simulink model "TosAeb"  (R2021a)
settings: Solver=FixedStepDiscrete  StartTime=0.0  StopTime=inf  FixedStep=0.01  RelTol=1e-3
undefined symbols (not defined in the file or in its folder): ACCModSts, AccSts1, AEB_ObjectMovingOrientation, AEB_ObjectMovingState, CN_BTCActive, CN_CurrentGearR, CN_CurrentGearR_DHT, CN_PTCActive, CN_VDCActive, CN_VSC_EspOff, CN_VSC_FCW_AEB_Error, CN_VSC_FCW_AEB_NoDispay, CN_VSC_FCW_AEB_PerfmDgdtn, CN_VSC_FCW_AEB_TempUnAvlbl, CN_VSC_VehStandstill, CNaccelAvoidMax, CNaccelAvoidMin, CNbrakePedalPress, CNcomfortStrLeftAccelMax, CNcomfortStrLeftAccelMin, CNcomfortStrRightAccelMax, CNcomfortStrRightAccelMin, CNDrvDoorOpen, CNDrvModeEco, CNDrvModeNorm, CNDrvModeOther, CNDrvModeSport, CNDrvSBRreminder, CNNull, CNobjInvalidID, CNPassDoorOpen, CNPassSBRreminder, CNPEBSAccelNegInf, CNPEBSAccelNull, CNPEBSTimeInf, CNPEBSTimeNull, CNSTOPMAX, CNSTOPNULL, CNsubjWidth, CNSwtOFF, CNSwtON, CNTTCMAX, CNTTCNULL, CNVehConfigAEBCycNone, CNVehConfigAEBNone, CNVehConfigAEBPedNone, CNVehConfigFCWNone, CNVehConfigJANone, CollisionSeverity, FALSE, FCWSnvty, JACycObjCalcInfoDefaultParams, JAPedObjCalcInfoDefaultParams, JAVehObjCalcInfoDefaultParams, latCycObjCalcInfoDefaultParams, latPedObjCalcInfoDefaultParams, longObjCalcInfoDefaultParams, ModSts, movingStatus, ObjectType ... (+1112)
libraries: simulink x3031, AEBSFunctionLib x50, Ta_Library x33, AEBSStateMachineLib x23, Mylibrary x12, simulink_extras x7, ObjSelect_lib x3, dspstat3 x2
(part 98 of 102)

== stateflow chart TosAeb/FcwAebJa_Step_sys/ALG_AEBSystem/ALG_AEBController/AEB_LongTgtInfoCalc/LongObjCalcInfoCalc/AEBS_LongCollisionAssesApp/TTCCalc/TTCCalc/Situation2/SloveTTCQuadraticEquation/AccelRelUnequalZero/DeltaGreaterEqualZero/RawTTCCalcu ==
  (default) -> (junction 11)
  (junction 11) -> (junction 12) [order 1] : [TTC1 < 0 && TTC2 < 0]
  (junction 11) -> (junction 13) [order 2]
  (junction 12) -> (junction 17) : {
      | 	TTC = CNNull;
      | }
  (junction 13) -> (junction 14) [order 1] : [TTC1 > 0 && TTC2 > 0]
  (junction 13) -> (junction 15) [order 2] : {
      | 	TTC = max(TTC1,TTC2);
      | }
  (junction 14) -> (junction 16) : {
      | 	TTC = min(TTC1,TTC2);
      | }
  (junction 16) -> (junction 15)
  (junction 17) -> (junction 16)
  (junction 15) -> (junction 18)
  data: TTC1 input; TTC2 input; TTC output; CNNull constant double

== stateflow chart TosAeb/FcwAebJa_Step_sys/ALG_AEBSystem/ALG_AEBController/AEB_LongTgtInfoCalc/LongObjCalcInfoCalc/AEBS_LongCollisionAssesApp/TTCCalc/TTCCalc/Situation3/SloveTTCQuadraticEquation/AccelRelEqualsZero/Chart ==
  state tempTTC = TTCCalc(distRel,velRel) [function]
  (default) -> (junction 17)
  (junction 17) -> (junction 18) [order 1] : [velRel >= 0 && distRel >= 0]
  (junction 17) -> (junction 19) [order 2]
  (junction 18) -> (junction 23) : {
      | 	TTC = CNSTOPMAX;
      | }
  (junction 19) -> (junction 20) [order 1] : [velRel <= 0 && distRel <= 0]
  (junction 19) -> (junction 21) [order 2] : {
      | 	 TTC = TTCCalc(distRel,velRel);
      | }
  (junction 20) -> (junction 22) : {
      | 	TTC = CNNull;
      | }
  (junction 22) -> (junction 21)
  (junction 23) -> (junction 22)
  (junction 21) -> (junction 24)
  data: distRel function_input; velRel function_input; tempTTC function_output; TTC output; CNSTOPMAX parameter; CNNull parameter; distRel input; velRel input

== stateflow chart TosAeb/FcwAebJa_Step_sys/ALG_AEBSystem/ALG_AEBController/AEB_LongTgtInfoCalc/LongObjCalcInfoCalc/AEBS_LongCollisionAssesApp/TTCCalc/TTCCalc/Situation3/SloveTTCQuadraticEquation/AccelRelUnequalZero/DeltaGreaterEqualZero/RawTTCCalcu ==
  (default) -> (junction 11)
  (junction 11) -> (junction 12) [order 1] : [TTC1 < 0 && TTC2 < 0]
  (junction 11) -> (junction 13) [order 2]
  (junction 12) -> (junction 17) : {
      | 	TTC = CNNull;
      | }
  (junction 13) -> (junction 14) [order 1] : [TTC1 > 0 && TTC2 > 0]
  (junction 13) -> (junction 15) [order 2] : {
      | 	TTC = max(TTC1,TTC2);
      | }
  (junction 14) -> (junction 16) : {
      | 	TTC = min(TTC1,TTC2);
      | }
  (junction 16) -> (junction 15)
  (junction 17) -> (junction 16)
  (junction 15) -> (junction 18)
  data: TTC1 input; TTC2 input; TTC output; CNNull constant double

== stateflow chart TosAeb/FcwAebJa_Step_sys/ALG_AEBSystem/ALG_AEBController/AEB_LongTgtInfoCalc/LongObjCalcInfoCalc/AEBS_LongCollisionAssesApp/TTCCalc/TTCCalc/SloveTTCQuadraticEquation/AccelRelEqualsZero/Chart ==
  state tempTTC = TTCCalc(distRel,velRel) [function]
  (default) -> (junction 17)
  (junction 17) -> (junction 18) [order 1] : [velRel >= 0 && distRel >= 0]
  (junction 17) -> (junction 19) [order 2]
  (junction 18) -> (junction 23) : {
      | 	TTC = CNSTOPMAX;
      | }
  (junction 19) -> (junction 20) [order 1] : [velRel <= 0 && distRel <= 0]
  (junction 19) -> (junction 21) [order 2] : {
      | 	 TTC = TTCCalc(distRel,velRel);
      | }
  (junction 20) -> (junction 22) : {
      | 	TTC = CNNull;
      | }
  (junction 22) -> (junction 21)
  (junction 23) -> (junction 22)
  (junction 21) -> (junction 24)
  data: distRel function_input; velRel function_input; tempTTC function_output; TTC output; CNSTOPMAX parameter; CNNull parameter; distRel input; velRel input

== stateflow chart TosAeb/FcwAebJa_Step_sys/ALG_AEBSystem/ALG_AEBController/AEB_LongTgtInfoCalc/LongObjCalcInfoCalc/AEBS_LongCollisionAssesApp/TTCCalc/TTCCalc/SloveTTCQuadraticEquation/AccelRelUnequalZero/DeltaGreaterEqualZero/RawTTCCalcu ==
  (default) -> (junction 11)
  (junction 11) -> (junction 12) [order 1] : [TTC1 < 0 && TTC2 < 0]
  (junction 11) -> (junction 13) [order 2]
  (junction 12) -> (junction 17) : {
      | 	TTC = CNNull;
      | }
  (junction 13) -> (junction 14) [order 1] : [TTC1 > 0 && TTC2 > 0]
  (junction 13) -> (junction 15) [order 2] : {
      | 	TTC = max(TTC1,TTC2);
      | }
  (junction 14) -> (junction 16) : {
      | 	TTC = min(TTC1,TTC2);
      | }
  (junction 16) -> (junction 15)
  (junction 17) -> (junction 16)
  (junction 15) -> (junction 18)
  data: TTC1 input; TTC2 input; TTC output; CNNull constant double

== stateflow chart TosAeb/FcwAebJa_Step_sys/ALG_AEBSystem/ALG_AEBController/AEB_LongTgtInfoCalc/LongObjCalcInfoCalc/AEBS_LongCollisionAssesApp/TTCCalc/TTCCalc/TTCCalc ==
  (default) -> (junction 17)
  (junction 17) -> (junction 18) [order 1] : [rawTTC < tEgoStop &&...
      | rawTTC < tObjStop]
  (junction 17) -> (junction 19) [order 2]
  (junction 18) -> (junction 23) : {TTC = rawTTC;}
  (junction 19) -> (junction 20) [order 1] : [rawTTC > tEgoStop &&...
      |  rawTTC < tObjStop]
  (junction 19) -> (junction 26) [order 2]
  (junction 20) -> (junction 22) : {
      | 	send(situation2);
      | 	TTC = TTCIn1;
      | }
  (junction 26) -> (junction 27) [order 1] : [rawTTC > tObjStop &&...
      |  rawTTC < tEgoStop]
  (junction 26) -> (junction 21) [order 2] : {TTC = CNTTCMAX;}
  (junction 27) -> (junction 29) : {
      | 	send(situation3);
      | 	TTC = TTCIn2;
      | }
  (junction 29) -> (junction 21)
  (junction 22) -> (junction 29)
  (junction 23) -> (junction 22)
  (junction 21) -> (junction 25)
  data: tEgoStop input; tObjStop input; rawTTC input; TTC output single; CNTTCMAX parameter single; TTCIn1 input; TTCIn2 input
  events: situation2 output FUNCTION_CALL_EVENT; situation3 output FUNCTION_CALL_EVENT

== stateflow chart TosAeb/FcwAebJa_Step_sys/ALG_AEBSystem/ALG_AEBController/AEB_LongTgtInfoCalc/LongObjCalcInfoCalc/AEBS_LongCollisionAssesApp/TTCCalc/TTCCalc/tEgoStopCalc/StopTimeFlow ==
  state temptStop = tStopFuncCalc(velRel,accelRel) [function]
  (default) -> (junction 2)
  (junction 2) -> (junction 3) [order 1] : [accelRel == 0]
  (junction 2) -> (junction 14) [order 2]
  (junction 3) -> (junction 5)
  (junction 14) -> (junction 16) [order 1] : [(accelRel > 0 && velRel <= 0)||...
      | (accelRel < 0 && velRel >= 0)]
  (junction 5) -> (junction 7) [order 1] : [velRel == 0]
  (junction 14) -> (junction 20) [order 2] : {
      | 	tStop = CNSTOPMAX;
      |  }
  (junction 16) -> (junction 18) : {
      |
      | 	tStop = tStopFuncCalc(velRel,accelRel);
      | }
  (junction 5) -> (junction 11) [order 2] : {
      | 	tStop = CNSTOPMAX;
      | }
  (junction 7) -> (junction 9) : {
      | 	tStop = CNNull;
      | }
  (junction 18) -> (junction 20)
  (junction 11) -> (junction 18)
  (junction 9) -> (junction 11)
  (junction 20) -> (junction 24)
  data: velRel function_input; accelRel function_input; temptStop function_output; tStop output; CNNull parameter double; CNSTOPMAX parameter double; accelRel input; velRel input

== stateflow chart TosAeb/FcwAebJa_Step_sys/ALG_AEBSystem/ALG_AEBController/AEB_LongTgtInfoCalc/LongObjCalcInfoCalc/AEBS_LongCollisionAssesApp/TTCCalc/TTCCalc/tObjStopCalc/StopTimeFlow ==
  state temptStop = tStopFuncCalc(velRel,accelRel) [function]
  (default) -> (junction 2)
  (junction 2) -> (junction 3) [order 1] : [accelRel == 0]
  (junction 2) -> (junction 14) [order 2]
  (junction 3) -> (junction 5)
  (junction 14) -> (junction 16) [order 1] : [(accelRel > 0 && velRel <= 0)||...
      | (accelRel < 0 && velRel >= 0)]
  (junction 5) -> (junction 7) [order 1] : [velRel == 0]
  (junction 14) -> (junction 20) [order 2] : {
      | 	tStop = CNSTOPMAX;
      |  }
  (junction 16) -> (junction 18) : {
      |
      | 	tStop = tStopFuncCalc(velRel,accelRel);
      | }
  (junction 5) -> (junction 11) [order 2] : {
      | 	tStop = CNSTOPMAX;
      | }
  (junction 7) -> (junction 9) : {
      | 	tStop = CNNull;
      | }
  (junction 18) -> (junction 20)
  (junction 11) -> (junction 18)
  (junction 9) -> (junction 11)
  (junction 20) -> (junction 24)
  data: velRel function_input; accelRel function_input; temptStop function_output; tStop output; CNNull parameter double; CNSTOPMAX parameter double; accelRel input; velRel input

== stateflow chart TosAeb/FcwAebJa_Step_sys/ALG_AEBSystem/ALG_AEBController/AEB_LongTgtInfoCalc/LongObjCalcInfoCalc/AEBS_LongCollisionAssesApp/collisionSeverityHighCalc/collisionSeverityHighCalc1/CollisionAssessHighCalcVersion3/CollisionAssessHighCalc1/CalcBrakePhase1/tEgoStopCalc/tStopCalc/StopTimeFlow ==
  state temptStop = tStopFuncCalc(velRel,accelRel) [function]
  (default) -> (junction 2)
  (junction 2) -> (junction 3) [order 1] : [accelRel == 0]
  (junction 2) -> (junction 14) [order 2]
  (junction 3) -> (junction 5)
  (junction 14) -> (junction 16) [order 1] : [(accelRel > 0 && velRel <= 0)||...
      | (accelRel < 0 && velRel >= 0)]
  (junction 5) -> (junction 7) [order 1] : [velRel == 0]
  (junction 14) -> (junction 20) [order 2] : {
      | 	tStop = CNSTOPMAX;
      |  }
  (junction 16) -> (junction 18) : {
      |
      | 	tStop = tStopFuncCalc(velRel,accelRel);
      | }
  (junction 5) -> (junction 11) [order 2] : {
      | 	tStop = CNSTOPMAX;
      | }
  (junction 7) -> (junction 9) : {
      | 	tStop = CNNull;
      | }
  (junction 18) -> (junction 20)
  (junction 11) -> (junction 18)
  (junction 9) -> (junction 11)
  (junction 20) -> (junction 24)
  data: velRel function_input; accelRel function_input; temptStop function_output; tStop output; CNNull parameter double; CNSTOPMAX parameter double; accelRel input; velRel input

== stateflow chart TosAeb/FcwAebJa_Step_sys/ALG_AEBSystem/ALG_AEBController/AEB_LongTgtInfoCalc/LongObjCalcInfoCalc/AEBS_LongCollisionAssesApp/collisionSeverityHighCalc/collisionSeverityHighCalc1/CollisionAssessHighCalcVersion3/CollisionAssessHighCalc1/CalcBrakePhase1/tObjStopCalc/tStopCalc/StopTimeFlow ==
  state temptStop = tStopFuncCalc(velRel,accelRel) [function]
  (default) -> (junction 2)
  (junction 2) -> (junction 3) [order 1] : [accelRel == 0]
  (junction 2) -> (junction 14) [order 2]
  (junction 3) -> (junction 5)
  (junction 14) -> (junction 16) [order 1] : [(accelRel > 0 && velRel <= 0)||...
      | (accelRel < 0 && velRel >= 0)]
  (junction 5) -> (junction 7) [order 1] : [velRel == 0]
  (junction 14) -> (junction 20) [order 2] : {
      | 	tStop = CNSTOPMAX;
      |  }
  (junction 16) -> (junction 18) : {
      |
      | 	tStop = tStopFuncCalc(velRel,accelRel);
      | }
  (junction 5) -> (junction 11) [order 2] : {
      | 	tStop = CNSTOPMAX;
      | }
  (junction 7) -> (junction 9) : {
      | 	tStop = CNNull;
      | }
  (junction 18) -> (junction 20)
  (junction 11) -> (junction 18)
  (junction 9) -> (junction 11)
  (junction 20) -> (junction 24)
  data: velRel function_input; accelRel function_input; temptStop function_output; tStop output; CNNull parameter double; CNSTOPMAX parameter double; accelRel input; velRel input

== stateflow chart TosAeb/FcwAebJa_Step_sys/ALG_AEBSystem/ALG_AEBController/AEB_LongTgtInfoCalc/LongObjCalcInfoCalc/AEBS_LongCollisionAssesApp/collisionSeverityHighCalc/collisionSeverityHighCalc1/CollisionAssessHighCalcVersion3/CollisionAssessHighCalc1/CalcBrakePhase1/timeEgovDeltaCalc/timeEgovDelata/StopTimeFlow ==
  state temptStop = tStopFuncCalc(velRel,accelRel) [function]
  (default) -> (junction 2)
  (junction 2) -> (junction 3) [order 1] : [accelRel == 0]
  (junction 2) -> (junction 14) [order 2]
  (junction 3) -> (junction 5)
  (junction 14) -> (junction 16) [order 1] : [(accelRel > 0 && velRel <= 0)||...
      | (accelRel < 0 && velRel >= 0)]
  (junction 5) -> (junction 7) [order 1] : [velRel == 0]
  (junction 14) -> (junction 20) [order 2] : {
      | 	tStop = CNSTOPMAX;
      |  }
  (junction 16) -> (junction 18) : {
      |
      | 	tStop = tStopFuncCalc(velRel,accelRel);
      | }
  (junction 5) -> (junction 11) [order 2] : {
      | 	tStop = CNSTOPMAX;
      | }
  (junction 7) -> (junction 9) : {
      | 	tStop = CNNull;
      | }
  (junction 18) -> (junction 20)
  (junction 11) -> (junction 18)
  (junction 9) -> (junction 11)
  (junction 20) -> (junction 24)
  data: velRel function_input; accelRel function_input; temptStop function_output; tStop output; CNNull parameter double; CNSTOPMAX parameter double; accelRel input; velRel input

== stateflow chart TosAeb/FcwAebJa_Step_sys/ALG_AEBSystem/ALG_AEBController/AEB_LongTgtInfoCalc/LongObjCalcInfoCalc/AEBS_LongCollisionAssesApp/collisionSeverityHighCalc/collisionSeverityHighCalc1/CollisionAssessHighCalcVersion3/CollisionAssessHighCalc1/CalcBrakePhase2/tEgoStopCalc/tStopCalc/StopTimeFlow ==
  state temptStop = tStopFuncCalc(velRel,accelRel) [function]
  (default) -> (junction 2)
  (junction 2) -> (junction 3) [order 1] : [accelRel == 0]
  (junction 2) -> (junction 14) [order 2]
  (junction 3) -> (junction 5)
  (junction 14) -> (junction 16) [order 1] : [(accelRel > 0 && velRel <= 0)||...
      | (accelRel < 0 && velRel >= 0)]
  (junction 5) -> (junction 7) [order 1] : [velRel == 0]
  (junction 14) -> (junction 20) [order 2] : {
      | 	tStop = CNSTOPMAX;
      |  }
  (junction 16) -> (junction 18) : {
      |
      | 	tStop = tStopFuncCalc(velRel,accelRel);
      | }
  (junction 5) -> (junction 11) [order 2] : {
      | 	tStop = CNSTOPMAX;
      | }
  (junction 7) -> (junction 9) : {
      | 	tStop = CNNull;
      | }
  (junction 18) -> (junction 20)
  (junction 11) -> (junction 18)
  (junction 9) -> (junction 11)
  (junction 20) -> (junction 24)
  data: velRel function_input; accelRel function_input; temptStop function_output; tStop output; CNNull parameter double; CNSTOPMAX parameter double; accelRel input; velRel input

== stateflow chart TosAeb/FcwAebJa_Step_sys/ALG_AEBSystem/ALG_AEBController/AEB_LongTgtInfoCalc/LongObjCalcInfoCalc/AEBS_LongCollisionAssesApp/collisionSeverityHighCalc/collisionSeverityHighCalc1/CollisionAssessHighCalcVersion3/CollisionAssessHighCalc1/CalcBrakePhase2/tObjStopCalc/tStopCalc/StopTimeFlow ==
  state temptStop = tStopFuncCalc(velRel,accelRel) [function]
  (default) -> (junction 2)
  (junction 2) -> (junction 3) [order 1] : [accelRel == 0]
  (junction 2) -> (junction 14) [order 2]
  (junction 3) -> (junction 5)
  (junction 14) -> (junction 16) [order 1] : [(accelRel > 0 && velRel <= 0)||...
      | (accelRel < 0 && velRel >= 0)]
  (junction 5) -> (junction 7) [order 1] : [velRel == 0]
  (junction 14) -> (junction 20) [order 2] : {
      | 	tStop = CNSTOPMAX;
      |  }
  (junction 16) -> (junction 18) : {
      |
      | 	tStop = tStopFuncCalc(velRel,accelRel);
      | }
  (junction 5) -> (junction 11) [order 2] : {
      | 	tStop = CNSTOPMAX;
      | }
  (junction 7) -> (junction 9) : {
      | 	tStop = CNNull;
      | }
  (junction 18) -> (junction 20)
  (junction 11) -> (junction 18)
  (junction 9) -> (junction 11)
  (junction 20) -> (junction 24)
  data: velRel function_input; accelRel function_input; temptStop function_output; tStop output; CNNull parameter double; CNSTOPMAX parameter double; accelRel input; velRel input

== stateflow chart TosAeb/FcwAebJa_Step_sys/ALG_AEBSystem/ALG_AEBController/AEB_LongTgtInfoCalc/LongObjCalcInfoCalc/AEBS_LongCollisionAssesApp/collisionSeverityHighCalc/collisionSeverityHighCalc1/CollisionAssessHighCalcVersion3/CollisionAssessHighCalc1/CalcBrakePhase2/timeEgovDeltaCalc/timeEgovDelata/StopTimeFlow ==
  state temptStop = tStopFuncCalc(velRel,accelRel) [function]
  (default) -> (junction 2)
  (junction 2) -> (junction 3) [order 1] : [accelRel == 0]
  (junction 2) -> (junction 14) [order 2]
  (junction 3) -> (junction 5)
  (junction 14) -> (junction 16) [order 1] : [(accelRel > 0 && velRel <= 0)||...
      | (accelRel < 0 && velRel >= 0)]
  (junction 5) -> (junction 7) [order 1] : [velRel == 0]
  (junction 14) -> (junction 20) [order 2] : {
      | 	tStop = CNSTOPMAX;
      |  }
  (junction 16) -> (junction 18) : {
      |
      | 	tStop = tStopFuncCalc(velRel,accelRel);
      | }
  (junction 5) -> (junction 11) [order 2] : {
      | 	tStop = CNSTOPMAX;
      | }
  (junction 7) -> (junction 9) : {
      | 	tStop = CNNull;
      | }
  (junction 18) -> (junction 20)
  (junction 11) -> (junction 18)
  (junction 9) -> (junction 11)
  (junction 20) -> (junction 24)
  data: velRel function_input; accelRel function_input; temptStop function_output; tStop output; CNNull parameter double; CNSTOPMAX parameter double; accelRel input; velRel input

== stateflow chart TosAeb/FcwAebJa_Step_sys/ALG_AEBSystem/ALG_AEBController/AEB_LongTgtInfoCalc/LongObjCalcInfoCalc/AEBS_LongCollisionAssesApp/collisionSeverityHighCalc/collisionSeverityHighCalc1/CollisionAssessHighCalcVersion3/CollisionAssessHighCalc1/CalcBrakePhase3/tEgoStopCalc/tStopCalc/StopTimeFlow ==
  state temptStop = tStopFuncCalc(velRel,accelRel) [function]
  (default) -> (junction 2)
  (junction 2) -> (junction 3) [order 1] : [accelRel == 0]
  (junction 2) -> (junction 14) [order 2]
  (junction 3) -> (junction 5)
  (junction 14) -> (junction 16) [order 1] : [(accelRel > 0 && velRel <= 0)||...
      | (accelRel < 0 && velRel >= 0)]
  (junction 5) -> (junction 7) [order 1] : [velRel == 0]
  (junction 14) -> (junction 20) [order 2] : {
      | 	tStop = CNSTOPMAX;
      |  }
  (junction 16) -> (junction 18) : {
      |
      | 	tStop = tStopFuncCalc(velRel,accelRel);
      | }
  (junction 5) -> (junction 11) [order 2] : {
      | 	tStop = CNSTOPMAX;
      | }
  (junction 7) -> (junction 9) : {
      | 	tStop = CNNull;
      | }
  (junction 18) -> (junction 20)
  (junction 11) -> (junction 18)
  (junction 9) -> (junction 11)
  (junction 20) -> (junction 24)
  data: velRel function_input; accelRel function_input; temptStop function_output; tStop output; CNNull parameter double; CNSTOPMAX parameter double; accelRel input; velRel input

== stateflow chart TosAeb/FcwAebJa_Step_sys/ALG_AEBSystem/ALG_AEBController/AEB_LongTgtInfoCalc/LongObjCalcInfoCalc/AEBS_LongCollisionAssesApp/collisionSeverityHighCalc/collisionSeverityHighCalc1/CollisionAssessHighCalcVersion3/CollisionAssessHighCalc1/CalcBrakePhase3/tObjStopCalc/tStopCalc/StopTimeFlow ==
  state temptStop = tStopFuncCalc(velRel,accelRel) [function]
  (default) -> (junction 2)
  (junction 2) -> (junction 3) [order 1] : [accelRel == 0]
  (junction 2) -> (junction 14) [order 2]
  (junction 3) -> (junction 5)
  (junction 14) -> (junction 16) [order 1] : [(accelRel > 0 && velRel <= 0)||...
      | (accelRel < 0 && velRel >= 0)]
  (junction 5) -> (junction 7) [order 1] : [velRel == 0]
  (junction 14) -> (junction 20) [order 2] : {
      | 	tStop = CNSTOPMAX;
      |  }
  (junction 16) -> (junction 18) : {
      |
      | 	tStop = tStopFuncCalc(velRel,accelRel);
      | }
  (junction 5) -> (junction 11) [order 2] : {
      | 	tStop = CNSTOPMAX;
      | }
  (junction 7) -> (junction 9) : {
      | 	tStop = CNNull;
      | }
  (junction 18) -> (junction 20)
  (junction 11) -> (junction 18)
  (junction 9) -> (junction 11)
  (junction 20) -> (junction 24)
  data: velRel function_input; accelRel function_input; temptStop function_output; tStop output; CNNull parameter double; CNSTOPMAX parameter double; accelRel input; velRel input

== stateflow chart TosAeb/FcwAebJa_Step_sys/ALG_AEBSystem/ALG_AEBController/AEB_LongTgtInfoCalc/LongObjCalcInfoCalc/AEBS_LongCollisionAssesApp/collisionSeverityHighCalc/collisionSeverityHighCalc1/CollisionAssessHighCalcVersion3/CollisionAssessHighCalc1/CalcBrakePhase3/timeEgovDeltaCalc/timeEgovDelata/StopTimeFlow ==
  state temptStop = tStopFuncCalc(velRel,accelRel) [function]
  (default) -> (junction 2)
  (junction 2) -> (junction 3) [order 1] : [accelRel == 0]
  (junction 2) -> (junction 14) [order 2]
  (junction 3) -> (junction 5)
  (junction 14) -> (junction 16) [order 1] : [(accelRel > 0 && velRel <= 0)||...
      | (accelRel < 0 && velRel >= 0)]
  (junction 5) -> (junction 7) [order 1] : [velRel == 0]
  (junction 14) -> (junction 20) [order 2] : {
      | 	tStop = CNSTOPMAX;
      |  }
  (junction 16) -> (junction 18) : {
      |
      | 	tStop = tStopFuncCalc(velRel,accelRel);
      | }
  (junction 5) -> (junction 11) [order 2] : {
      | 	tStop = CNSTOPMAX;
      | }
  (junction 7) -> (junction 9) : {
      | 	tStop = CNNull;
      | }
  (junction 18) -> (junction 20)
  (junction 11) -> (junction 18)
  (junction 9) -> (junction 11)
  (junction 20) -> (junction 24)
  data: velRel function_input; accelRel function_input; temptStop function_output; tStop output; CNNull parameter double; CNSTOPMAX parameter double; accelRel input; velRel input

== stateflow chart TosAeb/FcwAebJa_Step_sys/ALG_AEBSystem/ALG_AEBInput/JaObjInfoMap/JaObjInfoMap/ObjType_Subsystem/ObjectType ==
  (default) -> (junction 17)
  (junction 17) -> (junction 18) [order 1] : [ObjCar==1]
  (junction 17) -> (junction 19) [order 2]
  (junction 18) -> (junction 23) : {ObjType = 2;}
  (junction 19) -> (junction 20) [order 1] : [ObjTruck ==1]
  (junction 19) -> (junction 26) [order 2]
  (junction 20) -> (junction 22) : {
      | 	ObjType=3;
      | }
  (junction 26) -> (junction 27) [order 1] : [ObjPed==1]
  (junction 26) -> (junction 83) [order 2]
  (junction 27) -> (junction 29) : {
      | 	ObjType=6;
      | }
  (junction 83) -> (junction 85) [order 1] : [ObjMotor==1]
  (junction 83) -> (junction 91) [order 2]
  (junction 85) -> (junction 87) : {ObjType=4;}
  (junction 91) -> (junction 93) [order 1] : [ObjBicycle==1]
  (junction 91) -> (junction 100) [order 2]
  (junction 93) -> (junction 95) : {ObjType=5;}
  (junction 100) -> (junction 102) [order 1] : [ObjGeneral==1]
  (junction 100) -> (junction 21) [order 2] : {ObjType=1;}
  (junction 102) -> (junction 104) : {ObjType=7;}
  (junction 104) -> (junction 21)
  (junction 95) -> (junction 104)
  (junction 87) -> (junction 95)
  (junction 29) -> (junction 87)
  (junction 22) -> (junction 29)
  (junction 23) -> (junction 22)
  (junction 21) -> (junction 25)
  data: ObjCar input; ObjTruck input; ObjPed input; ObjType output uint8; ObjMotor input; ObjBicycle input; ObjGeneral input; Unknown input

== stateflow chart TosAeb/FcwAebJa_Step_sys/ALG_AEBSystem/ALG_AEBController/AEB_LongTgtInfoCalc/LongObjCalcInfoCalc/AEBS_LongCollisionAssesApp/collisionSeverityHighCalc/collisionSeverityHighCalc1/CollisionAssessHighCalcVersion3/CollisionAssessHighCalc2/CalcBrakePhase1/tEgoStopCalc/tStopCalc/StopTimeFlow ==
  state temptStop = tStopFuncCalc(velRel,accelRel) [function]
  (default) -> (junction 2)
  (junction 2) -> (junction 3) [order 1] : [accelRel == 0]
  (junction 2) -> (junction 14) [order 2]
  (junction 3) -> (junction 5)
  (junction 14) -> (junction 16) [order 1] : [(accelRel > 0 && velRel <= 0)||...
      | (accelRel < 0 && velRel >= 0)]
  (junction 5) -> (junction 7) [order 1] : [velRel == 0]
  (junction 14) -> (junction 20) [order 2] : {
      | 	tStop = CNSTOPMAX;
      |  }
  (junction 16) -> (junction 18) : {
      |
      | 	tStop = tStopFuncCalc(velRel,accelRel);
      | }
  (junction 5) -> (junction 11) [order 2] : {
      | 	tStop = CNSTOPMAX;
      | }
  (junction 7) -> (junction 9) : {
      | 	tStop = CNNull;
      | }
  (junction 18) -> (junction 20)
  (junction 11) -> (junction 18)
  (junction 9) -> (junction 11)
  (junction 20) -> (junction 24)
  data: velRel function_input; accelRel function_input; temptStop function_output; tStop output; CNNull parameter double; CNSTOPMAX parameter double; accelRel input; velRel input

== stateflow chart TosAeb/FcwAebJa_Step_sys/ALG_AEBSystem/ALG_AEBController/AEB_LongTgtInfoCalc/LongObjCalcInfoCalc/AEBS_LongCollisionAssesApp/collisionSeverityHighCalc/collisionSeverityHighCalc1/CollisionAssessHighCalcVersion3/CollisionAssessHighCalc2/CalcBrakePhase1/tObjStopCalc/tStopCalc/StopTimeFlow ==
  state temptStop = tStopFuncCalc(velRel,accelRel) [function]
  (default) -> (junction 2)
  (junction 2) -> (junction 3) [order 1] : [accelRel == 0]
  (junction 2) -> (junction 14) [order 2]
  (junction 3) -> (junction 5)
  (junction 14) -> (junction 16) [order 1] : [(accelRel > 0 && velRel <= 0)||...
      | (accelRel < 0 && velRel >= 0)]
  (junction 5) -> (junction 7) [order 1] : [velRel == 0]
  (junction 14) -> (junction 20) [order 2] : {
      | 	tStop = CNSTOPMAX;
      |  }
  (junction 16) -> (junction 18) : {
      |
      | 	tStop = tStopFuncCalc(velRel,accelRel);
      | }
  (junction 5) -> (junction 11) [order 2] : {
      | 	tStop = CNSTOPMAX;
      | }
  (junction 7) -> (junction 9) : {
      | 	tStop = CNNull;
      | }
  (junction 18) -> (junction 20)
  (junction 11) -> (junction 18)
  (junction 9) -> (junction 11)
  (junction 20) -> (junction 24)
  data: velRel function_input; accelRel function_input; temptStop function_output; tStop output; CNNull parameter double; CNSTOPMAX parameter double; accelRel input; velRel input

== stateflow chart TosAeb/FcwAebJa_Step_sys/ALG_AEBSystem/ALG_AEBController/AEB_LongTgtInfoCalc/LongObjCalcInfoCalc/AEBS_LongCollisionAssesApp/collisionSeverityHighCalc/collisionSeverityHighCalc1/CollisionAssessHighCalcVersion3/CollisionAssessHighCalc2/CalcBrakePhase1/timeEgovDelataCalc/timeEgovDelata/StopTimeFlow ==
  state temptStop = tStopFuncCalc(velRel,accelRel) [function]
  (default) -> (junction 2)
  (junction 2) -> (junction 3) [order 1] : [accelRel == 0]
  (junction 2) -> (junction 14) [order 2]
  (junction 3) -> (junction 5)
  (junction 14) -> (junction 16) [order 1] : [(accelRel > 0 && velRel <= 0)||...
      | (accelRel < 0 && velRel >= 0)]
  (junction 5) -> (junction 7) [order 1] : [velRel == 0]
  (junction 14) -> (junction 20) [order 2] : {
      | 	tStop = CNSTOPMAX;
      |  }
  (junction 16) -> (junction 18) : {
      |
      | 	tStop = tStopFuncCalc(velRel,accelRel);
      | }
  (junction 5) -> (junction 11) [order 2] : {
      | 	tStop = CNSTOPMAX;
      | }
  (junction 7) -> (junction 9) : {
      | 	tStop = CNNull;
      | }
  (junction 18) -> (junction 20)
  (junction 11) -> (junction 18)
  (junction 9) -> (junction 11)
  (junction 20) -> (junction 24)
  data: velRel function_input; accelRel function_input; temptStop function_output; tStop output; CNNull parameter double; CNSTOPMAX parameter double; accelRel input; velRel input

== stateflow chart TosAeb/FcwAebJa_Step_sys/ALG_AEBSystem/ALG_AEBController/AEB_LongTgtInfoCalc/LongObjCalcInfoCalc/AEBS_LongCollisionAssesApp/collisionSeverityHighCalc/collisionSeverityHighCalc1/CollisionAssessHighCalcVersion3/CollisionAssessHighCalc2/CalcBrakePhase2/tEgoStopCalc/tStopCalc/StopTimeFlow ==
  state temptStop = tStopFuncCalc(velRel,accelRel) [function]
  (default) -> (junction 2)
  (junction 2) -> (junction 3) [order 1] : [accelRel == 0]
  (junction 2) -> (junction 14) [order 2]
  (junction 3) -> (junction 5)
  (junction 14) -> (junction 16) [order 1] : [(accelRel > 0 && velRel <= 0)||...
      | (accelRel < 0 && velRel >= 0)]
  (junction 5) -> (junction 7) [order 1] : [velRel == 0]
  (junction 14) -> (junction 20) [order 2] : {
      | 	tStop = CNSTOPMAX;
      |  }
  (junction 16) -> (junction 18) : {
      |
      | 	tStop = tStopFuncCalc(velRel,accelRel);
      | }
  (junction 5) -> (junction 11) [order 2] : {
      | 	tStop = CNSTOPMAX;
      | }
  (junction 7) -> (junction 9) : {
      | 	tStop = CNNull;
      | }
  (junction 18) -> (junction 20)
  (junction 11) -> (junction 18)
  (junction 9) -> (junction 11)
  (junction 20) -> (junction 24)
  data: velRel function_input; accelRel function_input; temptStop function_output; tStop output; CNNull parameter double; CNSTOPMAX parameter double; accelRel input; velRel input

== stateflow chart TosAeb/FcwAebJa_Step_sys/ALG_AEBSystem/ALG_AEBController/AEB_LongTgtInfoCalc/LongObjCalcInfoCalc/AEBS_LongCollisionAssesApp/collisionSeverityHighCalc/collisionSeverityHighCalc1/CollisionAssessHighCalcVersion3/CollisionAssessHighCalc2/CalcBrakePhase2/tObjStopCalc/tStopCalc/StopTimeFlow ==
  state temptStop = tStopFuncCalc(velRel,accelRel) [function]
  (default) -> (junction 2)
  (junction 2) -> (junction 3) [order 1] : [accelRel == 0]
  (junction 2) -> (junction 14) [order 2]
  (junction 3) -> (junction 5)
  (junction 14) -> (junction 16) [order 1] : [(accelRel > 0 && velRel <= 0)||...
      | (accelRel < 0 && velRel >= 0)]
  (junction 5) -> (junction 7) [order 1] : [velRel == 0]
  (junction 14) -> (junction 20) [order 2] : {
      | 	tStop = CNSTOPMAX;
      |  }
  (junction 16) -> (junction 18) : {
      |
      | 	tStop = tStopFuncCalc(velRel,accelRel);
      | }
  (junction 5) -> (junction 11) [order 2] : {
      | 	tStop = CNSTOPMAX;
      | }
  (junction 7) -> (junction 9) : {
      | 	tStop = CNNull;
      | }
  (junction 18) -> (junction 20)
  (junction 11) -> (junction 18)
  (junction 9) -> (junction 11)
  (junction 20) -> (junction 24)
  data: velRel function_input; accelRel function_input; temptStop function_output; tStop output; CNNull parameter double; CNSTOPMAX parameter double; accelRel input; velRel input

== stateflow chart TosAeb/FcwAebJa_Step_sys/ALG_AEBSystem/ALG_AEBController/AEB_LongTgtInfoCalc/LongObjCalcInfoCalc/AEBS_LongCollisionAssesApp/collisionSeverityHighCalc/collisionSeverityHighCalc1/CollisionAssessHighCalcVersion3/CollisionAssessHighCalc2/CalcBrakePhase2/timeEgovDelataCalc/timeEgovDelata/StopTimeFlow ==
  state temptStop = tStopFuncCalc(velRel,accelRel) [function]
  (default) -> (junction 2)
  (junction 2) -> (junction 3) [order 1] : [accelRel == 0]
  (junction 2) -> (junction 14) [order 2]
  (junction 3) -> (junction 5)
  (junction 14) -> (junction 16) [order 1] : [(accelRel > 0 && velRel <= 0)||...
      | (accelRel < 0 && velRel >= 0)]
  (junction 5) -> (junction 7) [order 1] : [velRel == 0]
  (junction 14) -> (junction 20) [order 2] : {
      | 	tStop = CNSTOPMAX;
      |  }
  (junction 16) -> (junction 18) : {
      |
      | 	tStop = tStopFuncCalc(velRel,accelRel);
      | }
  (junction 5) -> (junction 11) [order 2] : {
      | 	tStop = CNSTOPMAX;
      | }
  (junction 7) -> (junction 9) : {
      | 	tStop = CNNull;
      | }
  (junction 18) -> (junction 20)
  (junction 11) -> (junction 18)
  (junction 9) -> (junction 11)
  (junction 20) -> (junction 24)
  data: velRel function_input; accelRel function_input; temptStop function_output; tStop output; CNNull parameter double; CNSTOPMAX parameter double; accelRel input; velRel input

== stateflow chart TosAeb/FcwAebJa_Step_sys/ALG_AEBSystem/ALG_AEBController/AEB_LongTgtInfoCalc/LongObjCalcInfoCalc/AEBS_LongCollisionAssesApp/collisionSeverityHighCalc/collisionSeverityHighCalc1/CollisionAssessHighCalcVersion3/CollisionAssessHighCalc2/CalcBrakePhase3/tEgoStopCalc/tStopCalc/StopTimeFlow ==
  state temptStop = tStopFuncCalc(velRel,accelRel) [function]
  (default) -> (junction 2)
  (junction 2) -> (junction 3) [order 1] : [accelRel == 0]
  (junction 2) -> (junction 14) [order 2]
  (junction 3) -> (junction 5)
  (junction 14) -> (junction 16) [order 1] : [(accelRel > 0 && velRel <= 0)||...
      | (accelRel < 0 && velRel >= 0)]
  (junction 5) -> (junction 7) [order 1] : [velRel == 0]
  (junction 14) -> (junction 20) [order 2] : {
      | 	tStop = CNSTOPMAX;
      |  }
  (junction 16) -> (junction 18) : {
      |
      | 	tStop = tStopFuncCalc(velRel,accelRel);
      | }
  (junction 5) -> (junction 11) [order 2] : {
      | 	tStop = CNSTOPMAX;
      | }
  (junction 7) -> (junction 9) : {
      | 	tStop = CNNull;
      | }
  (junction 18) -> (junction 20)
  (junction 11) -> (junction 18)
  (junction 9) -> (junction 11)
  (junction 20) -> (junction 24)
  data: velRel function_input; accelRel function_input; temptStop function_output; tStop output; CNNull parameter double; CNSTOPMAX parameter double; accelRel input; velRel input

== stateflow chart TosAeb/FcwAebJa_Step_sys/ALG_AEBSystem/ALG_AEBController/AEB_LongTgtInfoCalc/LongObjCalcInfoCalc/AEBS_LongCollisionAssesApp/collisionSeverityHighCalc/collisionSeverityHighCalc1/CollisionAssessHighCalcVersion3/CollisionAssessHighCalc2/CalcBrakePhase3/tObjStopCalc/tStopCalc/StopTimeFlow ==
  state temptStop = tStopFuncCalc(velRel,accelRel) [function]
  (default) -> (junction 2)
  (junction 2) -> (junction 3) [order 1] : [accelRel == 0]
  (junction 2) -> (junction 14) [order 2]
  (junction 3) -> (junction 5)
  (junction 14) -> (junction 16) [order 1] : [(accelRel > 0 && velRel <= 0)||...
      | (accelRel < 0 && velRel >= 0)]
  (junction 5) -> (junction 7) [order 1] : [velRel == 0]
  (junction 14) -> (junction 20) [order 2] : {
      | 	tStop = CNSTOPMAX;
      |  }
  (junction 16) -> (junction 18) : {
      |
      | 	tStop = tStopFuncCalc(velRel,accelRel);
      | }
  (junction 5) -> (junction 11) [order 2] : {
      | 	tStop = CNSTOPMAX;
      | }
  (junction 7) -> (junction 9) : {
      | 	tStop = CNNull;
      | }
  (junction 18) -> (junction 20)
  (junction 11) -> (junction 18)
  (junction 9) -> (junction 11)
  (junction 20) -> (junction 24)
  data: velRel function_input; accelRel function_input; temptStop function_output; tStop output; CNNull parameter double; CNSTOPMAX parameter double; accelRel input; velRel input

== stateflow chart TosAeb/FcwAebJa_Step_sys/ALG_AEBSystem/ALG_AEBController/AEB_LongTgtInfoCalc/LongObjCalcInfoCalc/AEBS_LongCollisionAssesApp/collisionSeverityHighCalc/collisionSeverityHighCalc1/CollisionAssessHighCalcVersion3/CollisionAssessHighCalc2/CalcBrakePhase3/timeEgovDelataCalc/timeEgovDelata/StopTimeFlow ==
  state temptStop = tStopFuncCalc(velRel,accelRel) [function]
  (default) -> (junction 2)
  (junction 2) -> (junction 3) [order 1] : [accelRel == 0]
  (junction 2) -> (junction 14) [order 2]
  (junction 3) -> (junction 5)
  (junction 14) -> (junction 16) [order 1] : [(accelRel > 0 && velRel <= 0)||...
      | (accelRel < 0 && velRel >= 0)]
  (junction 5) -> (junction 7) [order 1] : [velRel == 0]
  (junction 14) -> (junction 20) [order 2] : {
      | 	tStop = CNSTOPMAX;
      |  }
  (junction 16) -> (junction 18) : {
      |
      | 	tStop = tStopFuncCalc(velRel,accelRel);
      | }
  (junction 5) -> (junction 11) [order 2] : {
      | 	tStop = CNSTOPMAX;
      | }
  (junction 7) -> (junction 9) : {
      | 	tStop = CNNull;
      | }
  (junction 18) -> (junction 20)
  (junction 11) -> (junction 18)
  (junction 9) -> (junction 11)
  (junction 20) -> (junction 24)
  data: velRel function_input; accelRel function_input; temptStop function_output; tStop output; CNNull parameter double; CNSTOPMAX parameter double; accelRel input; velRel input

== stateflow chart TosAeb/FcwAebJa_Step_sys/ALG_AEBSystem/ALG_AEBController/AEB_LongTgtInfoCalc/LongObjCalcInfoCalc/AEBS_LongCollisionAssesApp/collisionSeverityHighCalc/collisionSeverityHighCalc2/collisionSeverityHighPhase1/Phase1TimeCalc/tObjStopCalc/StopTimeFlow ==
  state temptStop = tStopFuncCalc(velRel,accelRel) [function]
  (default) -> (junction 2)
  (junction 2) -> (junction 3) [order 1] : [accelRel == 0]
  (junction 2) -> (junction 14) [order 2]
  (junction 3) -> (junction 5)
  (junction 14) -> (junction 16) [order 1] : [(accelRel > 0 && velRel <= 0)||...
      | (accelRel < 0 && velRel >= 0)]
  (junction 5) -> (junction 7) [order 1] : [velRel == 0]
  (junction 14) -> (junction 20) [order 2] : {
      | 	tStop = CNSTOPMAX;
      |  }
  (junction 16) -> (junction 18) : {
      |
      | 	tStop = tStopFuncCalc(velRel,accelRel);
      | }
  (junction 5) -> (junction 11) [order 2] : {
      | 	tStop = CNSTOPMAX;
      | }
  (junction 7) -> (junction 9) : {
      | 	tStop = CNSTOPNULL;
      | }
  (junction 18) -> (junction 20)
  (junction 11) -> (junction 18)
  (junction 9) -> (junction 11)
  (junction 20) -> (junction 24)
  data: velRel function_input; accelRel function_input; temptStop function_output; tStop output; CNSTOPNULL parameter double; CNSTOPMAX parameter double; accelRel input; velRel input

== stateflow chart TosAeb/FcwAebJa_Step_sys/ALG_AEBSystem/ALG_AEBController/AEB_LongTgtInfoCalc/LongObjCalcInfoCalc/AEBS_LongCollisionAssesApp/collisionSeverityHighCalc/collisionSeverityHighCalc2/collisionSeverityHighPhase2/Phase2TimeCalc/tObjStopCalc/StopTimeFlow ==
  state temptStop = tStopFuncCalc(velRel,accelRel) [function]
  (default) -> (junction 2)
  (junction 2) -> (junction 3) [order 1] : [accelRel == 0]
  (junction 2) -> (junction 14) [order 2]
  (junction 3) -> (junction 5)
  (junction 14) -> (junction 16) [order 1] : [(accelRel > 0 && velRel <= 0)||...
      | (accelRel < 0 && velRel >= 0)]
  (junction 5) -> (junction 7) [order 1] : [velRel == 0]
  (junction 14) -> (junction 20) [order 2] : {
      | 	tStop = CNSTOPMAX;
      |  }
  (junction 16) -> (junction 18) : {
      |
      | 	tStop = tStopFuncCalc(velRel,accelRel);
      | }
  (junction 5) -> (junction 11) [order 2] : {
      | 	tStop = CNSTOPMAX;
      | }
  (junction 7) -> (junction 9) : {
      | 	tStop = CNSTOPNULL;
      | }
  (junction 18) -> (junction 20)
  (junction 11) -> (junction 18)
  (junction 9) -> (junction 11)
  (junction 20) -> (junction 24)
  data: velRel function_input; accelRel function_input; temptStop function_output; tStop output; CNSTOPNULL parameter double; CNSTOPMAX parameter double; accelRel input; velRel input

== stateflow chart TosAeb/FcwAebJa_Step_sys/ALG_AEBSystem/ALG_AEBController/AEB_LongTgtInfoCalc/LongObjCalcInfoCalc/AEBS_LongCollisionAssesApp/collisionSeverityHighCalc/collisionSeverityHighCalc2/collisionSeverityHighPhase3/Phase3TimeCalc/tBrakeConstCalc/tBrakeConstCalcFlow ==
  (default) -> (junction 2)
  (junction 2) -> (junction 3) [order 1] : [axAbsWorkPhase2Target < accelMaxReduce]
  (junction 2) -> (junction 13) [order 2]
  (junction 3) -> (junction 5)
  (junction 13) -> (junction 15) [order 1] : [subjVelWorkPhase2 > vxAbsWorkPhase2Target]
  (junction 13) -> (junction 19) [order 2] : {
      | 	tBrakeConst = CNSTOPNULL;
      | }
  (junction 15) -> (junction 26)
  (junction 5) -> (junction 7) [order 1] : [accelMaxReduce == 0]
  (junction 5) -> (junction 11) [order 2] : {
      | 	send(Stuaiton1);
      | 	tBrakeConst = tBrakeConstIn1;
      | }
  (junction 7) -> (junction 9) : {
      | 	tBrakeConst = CNPEBSTimeInf;
      | }
  (junction 26) -> (junction 28) [order 1] : [accelMaxReduce == axAbsWorkPhase2Target]
  (junction 26) -> (junction 17) [order 2] : {
      | 	send(Stuaiton2);
      | tBrakeConst = tBrakeConstIn2;
      | }
  (junction 28) -> (junction 30) : {
      | 	tBrakeConst = CNPEBSTimeInf;
      |
      | }
  (junction 17) -> (junction 19)
  (junction 30) -> (junction 17)
  (junction 11) -> (junction 30)
  (junction 9) -> (junction 11)
  (junction 19) -> (junction 24)
  data: accelMaxReduce input; subjVelWorkPhase2 input; vxAbsWorkPhase2Target input; tBrakeConst output; CNPEBSTimeInf parameter double; CNSTOPNULL parameter double; axAbsWorkPhase2Target input; tBrakeConstIn1 input; tBrakeConstIn2 input
  events: Stuaiton1 output FUNCTION_CALL_EVENT; Stuaiton2 output FUNCTION_CALL_EVENT

== stateflow chart TosAeb/FcwAebJa_Step_sys/ALG_AEBSystem/ALG_AEBController/AEB_LongTgtInfoCalc/LongObjCalcInfoCalc/AEBS_LongCollisionAssesApp/collisionSeverityHighCalc/collisionSeverityHighCalc2/collisionSeverityHighPhase3/Phase3TimeCalc/tObjStopCalc/StopTimeFlow ==
  state temptStop = tStopFuncCalc(velRel,accelRel) [function]
  (default) -> (junction 2)
  (junction 2) -> (junction 3) [order 1] : [accelRel == 0]
  (junction 2) -> (junction 14) [order 2]
  (junction 3) -> (junction 5)
  (junction 14) -> (junction 16) [order 1] : [(accelRel > 0 && velRel <= 0)||...
      | (accelRel < 0 && velRel >= 0)]
  (junction 5) -> (junction 7) [order 1] : [velRel == 0]
  (junction 14) -> (junction 20) [order 2] : {
      | 	tStop = CNSTOPMAX;
      |  }
  (junction 16) -> (junction 18) : {
      |
      | 	tStop = tStopFuncCalc(velRel,accelRel);
      | }
  (junction 5) -> (junction 11) [order 2] : {
      | 	tStop = CNSTOPMAX;
      | }
  (junction 7) -> (junction 9) : {
      | 	tStop = CNSTOPNULL;
      | }
  (junction 18) -> (junction 20)
  (junction 11) -> (junction 18)
  (junction 9) -> (junction 11)
  (junction 20) -> (junction 24)
  data: velRel function_input; accelRel function_input; temptStop function_output; tStop output; CNSTOPNULL parameter double; CNSTOPMAX parameter double; accelRel input; velRel input

== stateflow chart TosAeb/FcwAebJa_Step_sys/ALG_AEBSystem/ALG_AEBController/AEB_LongTgtInfoCalc/LongObjCalcInfoCalc/OverlapCalculation/OverlapRatioCalcFlow ==
  state overlapRatio = overlapRatioCalc(dyCriticalEgoObj,dyOverlapActual) [function]
  (default) -> (junction 2) : {
      | 	dyOverlapActual = dyEgo2Center - dyObject2Center;
      | }
  (junction 2) -> (junction 3) [order 1] : [dyOverlapActual == 0]
  (junction 2) -> (junction 7) [order 2] : {
      | 	dyOverlapActualAbs = abs(dyOverlapActual);
      | }
  (junction 3) -> (junction 5) : {
      | 	tempOverlapRatio = 1;
      | }
  (junction 7) -> (junction 9) [order 1] : [dyOverlapActualAbs > dyCriticalEgoObj ]
  (junction 7) -> (junction 13) [order 2] : {
      | 	tempOverlapRatio = overlapRatioCalc(dyCriticalEgoObj,dyOverlapActual);
      | }
  (junction 9) -> (junction 11) : {
      | 	tempOverlapRatio = 0;
      | }
  (junction 11) -> (junction 13)
  (junction 5) -> (junction 11)
  (junction 13) -> (junction 17) : {
      | 	overlapRatio = tempOverlapRatio;
      | }
  data: dyCriticalEgoObj function_input; dyOverlapActual function_input; overlapRatio function_output; dyCriticalEgoObj input; dyEgo2Center input; overlapRatio output; tempOverlapRatio local double; dyOverlapActualAbs local double; dyObject2Center input; dyOverlapActual local double

== matlab function TosAeb/AEB_Objsel/TA_Processor/PreProcessor/ObjectPrecessor/TAObjInfoCalc/For Each Subsystem/Info_Check1/Orientation_check/Orientation_Classify1 ==
  data: ID_Change input; Fus_ObjOrientation output; Fus_ObsType input; ObsID_u8 input; ObsMotionCategory_u8 input; ObsPedOrientation_u8 input; ObsPosX_s input; ObsPosY_s input; ObsVelX_s input; ObsVelY_s input; ObsVelXAbs_s input; ObsVelYAbs_s input; ObsCutInFlg_u8 input; ObsCutInSpd_s input; ObsCutInSpdValid_b input; ObsAngle_s input; ObsPosType_u8 input; ObsPosYDelay input
function Fus_ObjOrientation = fcn(ID_Change,Fus_ObsType,ObsID_u8,ObsMotionCategory_u8,ObsPedOrientation_u8,ObsPosX_s,ObsPosY_s,ObsVelX_s,ObsVelY_s,...
    ObsVelXAbs_s,ObsVelYAbs_s,ObsCutInFlg_u8,ObsCutInSpd_s,ObsCutInSpdValid_b,ObsAngle_s,ObsPosType_u8,ObsPosYDelay)
%Fus_ObjOrientation 目标运动方向，定义如下
% 0：unknown 1:Preceeding 2:Crossing_to_Left
% 3: Crossing_to_Right 4:Cut_in 5:Cut_Out
% 6: Oncoming 7: LTAP 8:RTAP
ObjOrientation = uint8(0);
%检查目标是否为有效目标
if ID_Change == true || ObsID_u8 == uint8(0)
    ObjOrientation = uint8(0);
else
    %目标类型为车辆时目标的运动方向划分
    if Fus_ObsType > uint8(0) && Fus_ObsType < uint8(9)
        %Preceding判断
        if ObsPosX_s > single(0) && abs(ObsPosY_s) <= single(10.5) && ObsVelXAbs_s >= single(-0.5) && abs(ObsVelYAbs_s) <= (abs(ObsVelXAbs_s)+0.5) && (abs(ObsAngle_s)<single(pi/4)||abs(ObsAngle_s)>single(0.75*pi)) &&...
                ((ObsMotionCategory_u8 >= uint8(2) && ObsMotionCategory_u8 <= uint8(7))|| ObsMotionCategory_u8 == uint8(12))&& ObsPosType_u8>= uint8(1) && ObsPosType_u8<= uint8(3)
            %cut_in cut_out 判断
            if (ObsMotionCategory_u8 == uint8(5)||ObsMotionCategory_u8 == uint8(6)) && ObsCutInFlg_u8 == uint8(1) && abs(ObsCutInSpd_s) > single(0) && ObsCutInSpdValid_b == true && abs(ObsVelYAbs_s)>single(0)
                ObjOrientation = uint8(4);
            elseif ObsMotionCategory_u8 == uint8(7) && abs(ObsPosYDelay) - abs(ObsPosY_s) > single(0)
                ObjOrientation = uint8(5);
            else
                ObjOrientation = uint8(1);
            end
            % Crossing_to_Left/Right判断
        elseif ObsPosX_s > single(0) && ObsVelYAbs_s>= single(0) && abs(ObsVelYAbs_s) >= abs((ObsVelXAbs_s)+0.5) && (abs(ObsAngle_s)>single(pi/4)&&abs(ObsAngle_s)<single(0.75*pi))&& ObsMotionCategory_u8 == uint8(12)
            if ObsVelY_s > single(0) && ObsPosType_u8 ~= uint8(7) && ObsPosYDelay - ObsPosY_s< single(0)
                ObjOrientation = uint8(2);
            elseif ObsVelY_s < single(0) && ObsPosType_u8 ~= uint8(8) && ObsPosYDelay - ObsPosY_s> single(0)
                ObjOrientation = uint8(3);
            end
            % Oncoming
        elseif ObsPosX_s > single(0) && abs(ObsPosY_s) <= single(10.5) && ObsVelXAbs_s<single(0) && abs(ObsVelYAbs_s) <= abs(ObsVelXAbs_s) && (abs(ObsAngle_s)<single(pi/4)||abs(ObsAngle_s)>single(0.75*pi)) &&...
                ObsMotionCategory_u8 == uint8(13) && ObsPosType_u8 >= uint8(4) && ObsPosType_u8<= uint8(6)
            ObjOrientation = uint8(6);
            % LTAP
        elseif ObsMotionCategory_u8 == uint8(9)
            ObjOrientation = uint8(7);
            % RTAP
        elseif ObsMotionCategory_u8 == uint8(10)
            ObjOrientation = uint8(8);
        else
            ObjOrientation = uint8(0);
        end
        %目标类型为行人或两轮车时目标的运动方向划分
    else
        if (ObsPedOrientation_u8 == uint8(1)||ObsPedOrientation_u8 == uint8(5)||ObsPedOrientation_u8 == uint8(8))&& ObsPosX_s > single(0)
            %Oncoming
            if ObsVelXAbs_s < single(-0.5) && abs(ObsVelYAbs_s) <= abs(ObsVelXAbs_s) && abs(ObsPosYDelay) - abs(ObsPosY_s) < single(1)
                ObjOrientation = uint8(6);
                %Crossing_to_Left/Right判断
            elseif ObsVelXAbs_s < single(-0.5) && (abs(ObsVelYAbs_s) >= (abs(ObsVelXAbs_s)+0.5) || abs(ObsPosYDelay) - abs(ObsPosY_s) >= single(1)) && ObsVelY_s >single(0) && ObsPosYDelay - ObsPosY_s < single(0)
                ObjOrientation = uint8(2);
            elseif ObsVelXAbs_s < single(-0.5) && (abs(ObsVelYAbs_s) >= (abs(ObsVelXAbs_s)+0.5) || abs(ObsPosYDelay) - abs(ObsPosY_s) >= single(1)) && ObsVelY_s <single(0) && ObsPosYDelay - ObsPosY_s > single(0)
                ObjOrientation = uint8(3);
            end
        elseif (ObsPedOrientation_u8 == uint8(2)||ObsPedOrientation_u8 == uint8(4)||ObsPedOrientation_u8 == uint8(7))&& ObsPosX_s > single(0)
            %Preceeding
            if ObsVelXAbs_s > single(-0.50) && abs(ObsVelYAbs_s) <= (abs(ObsVelXAbs_s)+0.5) && abs((ObsPosYDelay) - (ObsPosY_s)) < single(2)
                ObjOrientation = uint8(1);
                %Crossing_to_Left/Right判断
            elseif ObsVelXAbs_s < single(0) && (abs(ObsVelYAbs_s) >= (abs(ObsVelXAbs_s)-0.5) || abs((ObsPosYDelay) - (ObsPosY_s)) >= single(1)) && ObsVelY_s >single(0) && ObsPosYDelay - ObsPosY_s > single(0)
                ObjOrientation = uint8(2);
            elseif ObsVelXAbs_s < single(0) && (abs(ObsVelYAbs_s) >= (abs(ObsVelXAbs_s)-0.5) || abs((ObsPosYDelay) - (ObsPosY_s)) >= single(1)) && ObsVelY_s <single(0) && ObsPosYDelay - ObsPosY_s < single(0)
                ObjOrientation = uint8(3);
            end
        elseif (ObsPedOrientation_u8 == uint8(3)||ObsPedOrientation_u8 == uint8(6))&& ObsPosX_s > single(0)
            %Crossing_to_Left/Right判断
            if ObsVelXAbs_s < single(2) && (abs(ObsVelYAbs_s) >= (abs(ObsVelXAbs_s)+0.5) || abs(ObsPosYDelay) - abs(ObsPosY_s) >= single(1)) && ObsVelY_s >single(0) && ObsPosYDelay - ObsPosY_s < single(0)
                ObjOrientation = uint8(2);
            elseif ObsVelXAbs_s < single(2) && (abs(ObsVelYAbs_s) >= (abs(ObsVelXAbs_s)+0.5) || abs(ObsPosYDelay) - abs(ObsPosY_s) >= single(1)) && ObsVelY_s <single(0) && ObsPosYDelay - ObsPosY_s > single(0)
                ObjOrientation = uint8(3);
            end
        else
            ObjOrientation = uint8(0);
        end
    end
end
Fus_ObjOrientation = ObjOrientation;
end

== stateflow chart TosAeb/FcwAebJa_Step_sys/ALG_AEBSystem/ALG_AEBController/AEB_FuncCondCalcApp/LongFunctionCondCalc/vehEBASuppAborCondCalc/vehEBASuppCondCalc/vehEBASituationRelActiveCondCalc/brakeReleventSuppCond/brakeReleventSuppCond_ESP/brkTriggerCondCalc1 ==
  state MaxDecel = brkAccelThesLookup(subjVel) [function]
  (default) -> (junction 2)
  (junction 2) -> (junction 3) [order 1] : [objMovingState == AEB_ObjectMovingState.Still]
  (junction 2) -> (junction 28) [order 2] : {
      | 	%brkAccelThes = temp_brkAccelThes1;
      | brkAccelThes =brkAccelThesLookup(subjVel);
      | }
  (junction 3) -> (junction 14)
  (junction 14) -> (junction 16) [order 1] : [predictiveAccelAvoid < objStandAccelAvoidPredictThes]
  (junction 14) -> (junction 24) [order 2] : {
      | 	brkAccelThes =...
      |  	objStandBrkAccelThesLow;
      | }
  (junction 16) -> (junction 11) : {
      | 	brkAccelThes = ...
      | 	objStandBrkAccelThesHigh;
      | }
  (junction 24) -> (junction 28)
  (junction 11) -> (junction 24)
  (junction 28) -> (junction 31)
  (junction 31) -> (junction 48) [order 1] : [
      | 	brakePedalStatus == true && ...
      | 	ESPmasterCylindBrakePress > masterCylindBrkPressThes && ...
      | 	subjLongAccelFromSensor < brkAccelThes && ...
      | 	subjLongAccel < subjLongAccelThes
      | ]
  (junction 31) -> (junction 52) [order 2] : {
      | 	brkTriggerCondFulfilled = false;
      | }
  (junction 48) -> (junction 50) : {
      | 	brkTriggerCondFulfilled = true;
      | }
  (junction 50) -> (junction 52)
  (junction 52) -> (junction 55)
  data: subjVel function_input single; MaxDecel function_output; predictiveAccelAvoid input; objMovingState input; subjVel input; brkTriggerCondFulfilled output boolean; ESPmasterCylindBrakePress input; brakePedalStatus input; subjLongAccelFromSensor input; subjLongAccel input; masterCylindBrkPressThes input; objStandAccelAvoidPredictThes input; objStandBrkAccelThesHigh input; objStandBrkAccelThesLow input; subjLongAccelThes input; brkAccelThes local single

== stateflow chart TosAeb/FcwAebJa_Step_sys/ALG_AEBSystem/ALG_AEBController/AEB_VehicleStateCalcApp/FuncErrorStateJudge/FCW_AEB_JAFctStsCalc ==
  note: 0x00:No display
      | 0x01:error
      | 0x02:FCW/AEB_V temporarily unavailable
      | 0x03:FCW/AEB_V Performance degradation
  (default) -> (junction 80)
  (junction 80) -> (junction 82) [order 1] : [FCW_AEB_ErrorState == TRUE]
  (junction 80) -> (junction 84) [order 2]
  (junction 82) -> (junction 5) : {
      | 	FCW_AEB_JAFctSts = ...
      | CN_VSC_FCW_AEB_Error;
      | }
  (junction 84) -> (junction 92) [order 1] : [EspFuncOffSts == CN_VSC_EspOff]
  (junction 84) -> (junction 90) [order 2]
  (junction 92) -> (junction 88) : {
      | 	FCW_AEB_JAFctSts = ...
      | CN_VSC_FCW_AEB_PerfmDgdtn;
      | }
  (junction 90) -> (junction 86) [order 1] : [TrailerSts == TRUE]
  (junction 90) -> (junction 98) [order 2] : {
      | 	FCW_AEB_JAFctSts = ...
      | CN_VSC_FCW_AEB_NoDispay;
      | }
  (junction 86) -> (junction 94) : {
      | 	FCW_AEB_JAFctSts = ...
      | CN_VSC_FCW_AEB_TempUnAvlbl;
      | }
  (junction 94) -> (junction 98)
  (junction 88) -> (junction 94)
  (junction 5) -> (junction 88)
  (junction 98) -> (junction 103)
  data: FCW_AEB_JAFctSts output uint8; FCW_AEB_ErrorState input; CN_VSC_FCW_AEB_TempUnAvlbl parameter; EspFuncOffSts input; TrailerSts input; TRUE parameter; CN_VSC_FCW_AEB_PerfmDgdtn parameter; CN_VSC_FCW_AEB_NoDispay parameter; CN_VSC_FCW_AEB_Error parameter; CN_VSC_EspOff parameter
